simulink library "Garbage"  (R2023a)
settings: Solver=ode45  StartTime=0.0  StopTime=10.0  RelTol=1e-3  EnableMultiTasking=on
undefined symbols (not defined in the file or in its folder): c, ending_column_index, ending_row_index, framebuffer_16x1, framebuffer_2x2, framebuffer_4x4, framebuffer_8x8, HEIGHT, hot, POS_X, POS_Y, starting_column_index, starting_row_index, WIDTH
libraries: Memory_Blocks_Lib x459, simulink x235, simulink_extras x132, dspmtrx3 x116, Bit_Multiplexers_Lib x67, Bit_Demultiplexers_Lib x49, Byte_Multiplexers_Lib x40, Miscellaneous x38, Computer_Library x17, Decoders_Lib x14, commutil2 x12, Arithmetic_Operations_Lib x8
(part 7 of 26)

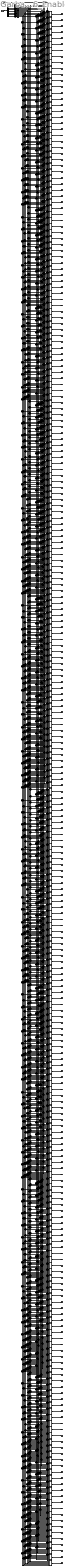
== system Garbage/Decoder_8_to_256_Enable ==
  Enable(1b) : Inport
  Addr(1B) : Inport  Port=2
  Lo`gical Operator{1,3,5..6,9} (5 alike) : Logic  Operator=NOT  IconShape=distinctive  AllPortsSameDT=off  OutDataTypeStr=boolean
  Logical Operator{1..81,84..111,113..146,148..243,246..262} (256 alike) : Logic  Inputs=9  IconShape=distinctive  AllPortsSameDT=off  OutDataTypeStr=boolean
  Logical Operator112 : Logic  Operator=NOT  IconShape=distinctive  AllPortsSameDT=off  OutDataTypeStr=boolean
  Logical Operator82 : Logic  Operator=NOT  IconShape=distinctive  AllPortsSameDT=off  OutDataTypeStr=boolean
  Logical Operator83 : Logic  Operator=NOT  IconShape=distinctive  AllPortsSameDT=off  OutDataTypeStr=boolean
  xyz1 : BusSelector  OutputSignals=signal1,signal2,signal3,signal4,signal5,signal6,signal7,signal8
  Select_255(1b) : Outport
  Select_254(1b) : Outport  Port=2
  Select_253(1b) : Outport  Port=3
  Select_252(1b) : Outport  Port=4
  Select_251(1b) : Outport  Port=5
  Select_250(1b) : Outport  Port=6
  Select_249(1b) : Outport  Port=7
  Select_248(1b) : Outport  Port=8
  Select_247(1b) : Outport  Port=9
  Select_246(1b) : Outport  Port=10
  Select_245(1b) : Outport  Port=11
  Select_244(1b) : Outport  Port=12
  Select_243(1b) : Outport  Port=13
  Select_242(1b) : Outport  Port=14
  Select_241(1b) : Outport  Port=15
  Select_240(1b) : Outport  Port=16
  Select_239(1b) : Outport  Port=17
  Select_238(1b) : Outport  Port=18
  Select_237(1b) : Outport  Port=19
  Select_236(1b) : Outport  Port=20
  Select_235(1b) : Outport  Port=21
  Select_234(1b) : Outport  Port=22
  Select_233(1b) : Outport  Port=23
  Select_232(1b) : Outport  Port=24
  Select_231(1b) : Outport  Port=25
  Select_230(1b) : Outport  Port=26
  Select_229(1b) : Outport  Port=27
  Select_228(1b) : Outport  Port=28
  Select_227(1b) : Outport  Port=29
  Select_226(1b) : Outport  Port=30
  Select_225(1b) : Outport  Port=31
  Select_224(1b) : Outport  Port=32
  Select_223(1b) : Outport  Port=33
  Select_222(1b) : Outport  Port=34
  Select_221(1b) : Outport  Port=35
  Select_220(1b) : Outport  Port=36
  Select_219(1b) : Outport  Port=37
  Select_218(1b) : Outport  Port=38
  Select_217(1b) : Outport  Port=39
  Select_216(1b) : Outport  Port=40
  Select_215(1b) : Outport  Port=41
  Select_214(1b) : Outport  Port=42
  Select_213(1b) : Outport  Port=43
  Select_212(1b) : Outport  Port=44
  Select_211(1b) : Outport  Port=45
  Select_210(1b) : Outport  Port=46
  Select_209(1b) : Outport  Port=47
  Select_208(1b) : Outport  Port=48
  Select_207(1b) : Outport  Port=49
  Select_206(1b) : Outport  Port=50
  Select_205(1b) : Outport  Port=51
  Select_204(1b) : Outport  Port=52
  Select_203(1b) : Outport  Port=53
  Select_202(1b) : Outport  Port=54
  Select_201(1b) : Outport  Port=55
  Select_200(1b) : Outport  Port=56
  Select_199(1b) : Outport  Port=57
  Select_198(1b) : Outport  Port=58
  Select_197(1b) : Outport  Port=59
  Select_196(1b) : Outport  Port=60
  Select_195(1b) : Outport  Port=61
  Select_194(1b) : Outport  Port=62
  Select_193(1b) : Outport  Port=63
  Select_192(1b) : Outport  Port=64
  Select_191(1b) : Outport  Port=65
  Select_190(1b) : Outport  Port=66
  Select_189(1b) : Outport  Port=67
  Select_188(1b) : Outport  Port=68
  Select_187(1b) : Outport  Port=69
  Select_186(1b) : Outport  Port=70
  Select_185(1b) : Outport  Port=71
  Select_184(1b) : Outport  Port=72
  Select_183(1b) : Outport  Port=73
  Select_182(1b) : Outport  Port=74
  Select_181(1b) : Outport  Port=75
  Select_180(1b) : Outport  Port=76
  Select_179(1b) : Outport  Port=77
  Select_178(1b) : Outport  Port=78
  Select_177(1b) : Outport  Port=79
  Select_176(1b) : Outport  Port=80
  Select_175(1b) : Outport  Port=81
  Select_174(1b) : Outport  Port=82
  Select_173(1b) : Outport  Port=83
  Select_172(1b) : Outport  Port=84
  Select_171(1b) : Outport  Port=85
  Select_170(1b) : Outport  Port=86
  Select_169(1b) : Outport  Port=87
  Select_168(1b) : Outport  Port=88
  Select_167(1b) : Outport  Port=89
  Select_166(1b) : Outport  Port=90
  Select_165(1b) : Outport  Port=91
  Select_164(1b) : Outport  Port=92
  Select_163(1b) : Outport  Port=93
  Select_162(1b) : Outport  Port=94
  Select_161(1b) : Outport  Port=95
  Select_160(1b) : Outport  Port=96
  Select_159(1b) : Outport  Port=97
  Select_158(1b) : Outport  Port=98
  Select_157(1b) : Outport  Port=99
  Select_156(1b) : Outport  Port=100
  Select_155(1b) : Outport  Port=101
  Select_154(1b) : Outport  Port=102
  Select_153(1b) : Outport  Port=103
  Select_152(1b) : Outport  Port=104
  Select_151(1b) : Outport  Port=105
  Select_150(1b) : Outport  Port=106
  Select_149(1b) : Outport  Port=107
  Select_148(1b) : Outport  Port=108
  Select_147(1b) : Outport  Port=109
  Select_146(1b) : Outport  Port=110
  Select_145(1b) : Outport  Port=111
  Select_144(1b) : Outport  Port=112
  Select_143(1b) : Outport  Port=113
  Select_142(1b) : Outport  Port=114
  Select_141(1b) : Outport  Port=115
  Select_140(1b) : Outport  Port=116
  Select_139(1b) : Outport  Port=117
  Select_138(1b) : Outport  Port=118
  Select_137(1b) : Outport  Port=119
  Select_136(1b) : Outport  Port=120
  Select_135(1b) : Outport  Port=121
  Select_134(1b) : Outport  Port=122
  Select_133(1b) : Outport  Port=123
  Select_132(1b) : Outport  Port=124
  Select_131(1b) : Outport  Port=125
  Select_130(1b) : Outport  Port=126
  Select_129(1b) : Outport  Port=127
  Select_128(1b) : Outport  Port=128
  Select_127(1b) : Outport  Port=129
  Select_126(1b) : Outport  Port=130
  Select_125(1b) : Outport  Port=131
  Select_124(1b) : Outport  Port=132
  Select_123(1b) : Outport  Port=133
  Select_122(1b) : Outport  Port=134
  Select_121(1b) : Outport  Port=135
  Select_120(1b) : Outport  Port=136
  Select_119(1b) : Outport  Port=137
  Select_118(1b) : Outport  Port=138
  Select_117(1b) : Outport  Port=139
  Select_116(1b) : Outport  Port=140
  Select_115(1b) : Outport  Port=141
  Select_114(1b) : Outport  Port=142
  Select_113(1b) : Outport  Port=143
  Select_112(1b) : Outport  Port=144
  Select_111(1b) : Outport  Port=145
  Select_110(1b) : Outport  Port=146
  Select_109(1b) : Outport  Port=147
  Select_108(1b) : Outport  Port=148
  Select_107(1b) : Outport  Port=149
  Select_106(1b) : Outport  Port=150
  Select_105(1b) : Outport  Port=151
  Select_104(1b) : Outport  Port=152
  Select_103(1b) : Outport  Port=153
  Select_102(1b) : Outport  Port=154
  Select_101(1b) : Outport  Port=155
  Select_100(1b) : Outport  Port=156
  Select_099(1b) : Outport  Port=157
  Select_098(1b) : Outport  Port=158
  Select_097(1b) : Outport  Port=159
  Select_096(1b) : Outport  Port=160
  Select_095(1b) : Outport  Port=161
  Select_094(1b) : Outport  Port=162
  Select_093(1b) : Outport  Port=163
  Select_092(1b) : Outport  Port=164
  Select_091(1b) : Outport  Port=165
  Select_090(1b) : Outport  Port=166
  Select_089(1b) : Outport  Port=167
  Select_088(1b) : Outport  Port=168
  Select_087(1b) : Outport  Port=169
  Select_086(1b) : Outport  Port=170
  Select_085(1b) : Outport  Port=171
  Select_084(1b) : Outport  Port=172
  Select_083(1b) : Outport  Port=173
  Select_082(1b) : Outport  Port=174
  Select_081(1b) : Outport  Port=175
  Select_080(1b) : Outport  Port=176
  Select_079(1b) : Outport  Port=177
  Select_078(1b) : Outport  Port=178
  Select_077(1b) : Outport  Port=179
  Select_076(1b) : Outport  Port=180
  Select_075(1b) : Outport  Port=181
  Select_074(1b) : Outport  Port=182
  Select_073(1b) : Outport  Port=183
  Select_072(1b) : Outport  Port=184
  Select_071(1b) : Outport  Port=185
  Select_070(1b) : Outport  Port=186
  Select_069(1b) : Outport  Port=187
  Select_068(1b) : Outport  Port=188
  Select_067(1b) : Outport  Port=189
  Select_066(1b) : Outport  Port=190
  Select_065(1b) : Outport  Port=191
  Select_064(1b) : Outport  Port=192
  Select_063(1b) : Outport  Port=193
  Select_062(1b) : Outport  Port=194
  Select_061(1b) : Outport  Port=195
  Select_060(1b) : Outport  Port=196
  Select_059(1b) : Outport  Port=197
  Select_058(1b) : Outport  Port=198
  Select_057(1b) : Outport  Port=199
  Select_056(1b) : Outport  Port=200
  Select_055(1b) : Outport  Port=201
  Select_054(1b) : Outport  Port=202
  Select_053(1b) : Outport  Port=203
  Select_052(1b) : Outport  Port=204
  Select_051(1b) : Outport  Port=205
  Select_050(1b) : Outport  Port=206
  Select_049(1b) : Outport  Port=207
  Select_048(1b) : Outport  Port=208
  Select_047(1b) : Outport  Port=209
  Select_046(1b) : Outport  Port=210
  Select_045(1b) : Outport  Port=211
  Select_044(1b) : Outport  Port=212
  Select_043(1b) : Outport  Port=213
  Select_042(1b) : Outport  Port=214
  Select_041(1b) : Outport  Port=215
  Select_040(1b) : Outport  Port=216
  Select_039(1b) : Outport  Port=217
  Select_038(1b) : Outport  Port=218
  Select_037(1b) : Outport  Port=219
  Select_036(1b) : Outport  Port=220
  Select_035(1b) : Outport  Port=221
  Select_034(1b) : Outport  Port=222
  Select_033(1b) : Outport  Port=223
  Select_032(1b) : Outport  Port=224
  Select_031(1b) : Outport  Port=225
  Select_030(1b) : Outport  Port=226
  Select_029(1b) : Outport  Port=227
  Select_028(1b) : Outport  Port=228
  Select_027(1b) : Outport  Port=229
  Select_026(1b) : Outport  Port=230
  Select_025(1b) : Outport  Port=231
  Select_024(1b) : Outport  Port=232
  Select_023(1b) : Outport  Port=233
  Select_022(1b) : Outport  Port=234
  Select_021(1b) : Outport  Port=235
  Select_020(1b) : Outport  Port=236
  Select_019(1b) : Outport  Port=237
  Select_018(1b) : Outport  Port=238
  Select_017(1b) : Outport  Port=239
  Select_016(1b) : Outport  Port=240
  Select_015(1b) : Outport  Port=241
  Select_014(1b) : Outport  Port=242
  Select_013(1b) : Outport  Port=243
  Select_012(1b) : Outport  Port=244
  Select_011(1b) : Outport  Port=245
  Select_010(1b) : Outport  Port=246
  Select_009(1b) : Outport  Port=247
  Select_008(1b) : Outport  Port=248
  Select_007(1b) : Outport  Port=249
  Select_006(1b) : Outport  Port=250
  Select_005(1b) : Outport  Port=251
  Select_004(1b) : Outport  Port=252
  Select_003(1b) : Outport  Port=253
  Select_002(1b) : Outport  Port=254
  Select_001(1b) : Outport  Port=255
  Select_000(1b) : Outport  Port=256
  wires:
  Logical Operator16/1 -> Select_176(1b)/1
  Logical Operator15/1 -> Select_177(1b)/1
  Logical Operator14/1 -> Select_178(1b)/1
  Logical Operator13/1 -> Select_179(1b)/1
  Logical Operator12/1 -> Select_180(1b)/1
  Logical Operator11/1 -> Select_181(1b)/1
  Logical Operator10/1 -> Select_182(1b)/1
  Logical Operator9/1 -> Select_183(1b)/1
  Logical Operator8/1 -> Select_184(1b)/1
  Logical Operator7/1 -> Select_185(1b)/1
  Logical Operator6/1 -> Select_186(1b)/1
  Logical Operator4/1 -> Select_187(1b)/1
  Logical Operator1/1 -> Select_190(1b)/1
  Logical Operator2/1 -> Select_189(1b)/1
  Logical Operator3/1 -> Select_188(1b)/1
  Logical Operator63/1 -> Select_191(1b)/1
  Logical Operator26/1 -> Select_160(1b)/1
  Logical Operator25/1 -> Select_161(1b)/1
  Logical Operator24/1 -> Select_162(1b)/1
  Logical Operator21/1 -> Select_163(1b)/1
  Logical Operator20/1 -> Select_164(1b)/1
  Logical Operator19/1 -> Select_165(1b)/1
  Logical Operator18/1 -> Select_166(1b)/1
  Logical Operator34/1 -> Select_167(1b)/1
  Logical Operator33/1 -> Select_168(1b)/1
  Logical Operator32/1 -> Select_169(1b)/1
  Logical Operator30/1 -> Select_170(1b)/1
  Logical Operator29/1 -> Select_171(1b)/1
  Logical Operator17/1 -> Select_174(1b)/1
  Logical Operator27/1 -> Select_173(1b)/1
  Logical Operator28/1 -> Select_172(1b)/1
  Logical Operator31/1 -> Select_175(1b)/1
  Logical Operator42/1 -> Select_144(1b)/1
  Logical Operator41/1 -> Select_145(1b)/1
  Logical Operator40/1 -> Select_146(1b)/1
  Logical Operator39/1 -> Select_147(1b)/1
  Logical Operator38/1 -> Select_148(1b)/1
  Logical Operator37/1 -> Select_149(1b)/1
  Logical Operator36/1 -> Select_150(1b)/1
  Logical Operator50/1 -> Select_151(1b)/1
  Logical Operator49/1 -> Select_152(1b)/1
  Logical Operator48/1 -> Select_153(1b)/1
  Logical Operator46/1 -> Select_154(1b)/1
  Logical Operator45/1 -> Select_155(1b)/1
  Logical Operator35/1 -> Select_158(1b)/1
  Logical Operator43/1 -> Select_157(1b)/1
  Logical Operator44/1 -> Select_156(1b)/1
  Logical Operator47/1 -> Select_159(1b)/1
  Logical Operator58/1 -> Select_128(1b)/1
  Logical Operator57/1 -> Select_129(1b)/1
  Logical Operator56/1 -> Select_130(1b)/1
  Logical Operator55/1 -> Select_131(1b)/1
  Logical Operator54/1 -> Select_132(1b)/1
  Logical Operator53/1 -> Select_133(1b)/1
  Logical Operator52/1 -> Select_134(1b)/1
  Logical Operator67/1 -> Select_135(1b)/1
  Logical Operator66/1 -> Select_136(1b)/1
  Logical Operator65/1 -> Select_137(1b)/1
  Logical Operator62/1 -> Select_138(1b)/1
  Logical Operator61/1 -> Select_139(1b)/1
  Logical Operator51/1 -> Select_142(1b)/1
  Logical Operator59/1 -> Select_141(1b)/1
  Logical Operator60/1 -> Select_140(1b)/1
  Logical Operator64/1 -> Select_143(1b)/1
  xyz1/1 -> Logical Operator68/2, Logical Operator79/2, Logical Operator90/2, Logical Operator101/2, Logical Operator123/2, Logical Operator132/2, Logical Operator133/2, Logical Operator134/2, Logical Operator69/2, Logical Operator70/2, Logical Operator71/2, Logical Operator72/2, Logical Operator73/2, Logical Operator74/2, Logical Operator75/2, Logical Operator92/2, Logical Operator76/2, Logical Operator87/2, Logical Operator88/2, Logical Operator89/2, Logical Operator91/2, Logical Operator93/2, Logical Operator94/2, Logical Operator95/2, Logical Operator77/2, Logical Operator78/2, Logical Operator80/2, Logical Operator81/2, Logical Operator84/2, Logical Operator85/2, Logical Operator86/2, Logical Operator109/2, Logical Operator96/2, Logical Operator105/2, Logical Operator106/2, Logical Operator107/2, Logical Operator108/2, Logical Operator110/2, Logical Operator111/2, Logical Operator113/2, Logical Operator97/2, Logical Operator98/2, Logical Operator99/2, Logical Operator100/2, Logical Operator102/2, Logical Operator103/2, Logical Operator104/2, Logical Operator128/2, Logical Operator114/2, Logical Operator122/2, Logical Operator124/2, Logical Operator125/2, Logical Operator126/2, Logical Operator129/2, Logical Operator130/2, Logical Operator131/2, Logical Operator115/2, Logical Operator116/2, Logical Operator117/2, Logical Operator118/2, Logical Operator119/2, Logical Operator120/2, Logical Operator121/2, Logical Operator63/2, Logical Operator1/2, Logical Operator2/2, Logical Operator3/2, Logical Operator4/2, Logical Operator6/2, Logical Operator7/2, Logical Operator8/2, Logical Operator9/2, Logical Operator10/2, Logical Operator11/2, Logical Operator12/2, Logical Operator13/2, Logical Operator14/2, Logical Operator15/2, Logical Operator16/2, Logical Operator31/2, Logical Operator17/2, Logical Operator27/2, Logical Operator28/2, Logical Operator29/2, Logical Operator30/2, Logical Operator32/2, Logical Operator33/2, Logical Operator34/2, Logical Operator18/2, Logical Operator19/2, Logical Operator20/2, Logical Operator21/2, Logical Operator24/2, Logical Operator25/2, Logical Operator26/2, Logical Operator47/2, Logical Operator35/2, Logical Operator43/2, Logical Operator44/2, Logical Operator45/2, Logical Operator46/2, Logical Operator48/2, Logical Operator49/2, Logical Operator50/2, Logical Operator36/2, Logical Operator37/2, Logical Operator38/2, Logical Operator39/2, Logical Operator40/2, Logical Operator41/2, Logical Operator42/2, Logical Operator64/2, Logical Operator51/2, Logical Operator59/2, Logical Operator60/2, Logical Operator61/2, Logical Operator62/2, Logical Operator65/2, Logical Operator66/2, Logical Operator67/2, Logical Operator52/2, Logical Operator53/2, Logical Operator54/2, Logical Operator55/2, Logical Operator56/2, Logical Operator57/2, Logical Operator58/2, Logical Operator127/2, Lo`gical Operator9/1  "<signal1>"
  Logical Operator75/1 -> Select_240(1b)/1
  Logical Operator74/1 -> Select_241(1b)/1
  Logical Operator73/1 -> Select_242(1b)/1
  Logical Operator72/1 -> Select_243(1b)/1
  Logical Operator71/1 -> Select_244(1b)/1
  Logical Operator70/1 -> Select_245(1b)/1
  Logical Operator69/1 -> Select_246(1b)/1
  Logical Operator134/1 -> Select_247(1b)/1
  Logical Operator133/1 -> Select_248(1b)/1
  Logical Operator132/1 -> Select_249(1b)/1
  Logical Operator123/1 -> Select_250(1b)/1
  Logical Operator101/1 -> Select_251(1b)/1
  Logical Operator68/1 -> Select_254(1b)/1
  Logical Operator79/1 -> Select_253(1b)/1
  Logical Operator90/1 -> Select_252(1b)/1
  Logical Operator127/1 -> Select_255(1b)/1
  Logical Operator86/1 -> Select_224(1b)/1
  Logical Operator85/1 -> Select_225(1b)/1
  Logical Operator84/1 -> Select_226(1b)/1
  Logical Operator81/1 -> Select_227(1b)/1
  Logical Operator80/1 -> Select_228(1b)/1
  Logical Operator78/1 -> Select_229(1b)/1
  Logical Operator77/1 -> Select_230(1b)/1
  Logical Operator95/1 -> Select_231(1b)/1
  Logical Operator94/1 -> Select_232(1b)/1
  Logical Operator93/1 -> Select_233(1b)/1
  Logical Operator91/1 -> Select_234(1b)/1
  Logical Operator89/1 -> Select_235(1b)/1
  Logical Operator76/1 -> Select_238(1b)/1
  Logical Operator87/1 -> Select_237(1b)/1
  Logical Operator88/1 -> Select_236(1b)/1
  Logical Operator92/1 -> Select_239(1b)/1
  Logical Operator104/1 -> Select_208(1b)/1
  Logical Operator103/1 -> Select_209(1b)/1
  Logical Operator102/1 -> Select_210(1b)/1
  Logical Operator100/1 -> Select_211(1b)/1
  Logical Operator99/1 -> Select_212(1b)/1
  Logical Operator98/1 -> Select_213(1b)/1
  Logical Operator97/1 -> Select_214(1b)/1
  Logical Operator113/1 -> Select_215(1b)/1
  Logical Operator111/1 -> Select_216(1b)/1
  Logical Operator110/1 -> Select_217(1b)/1
  Logical Operator108/1 -> Select_218(1b)/1
  Logical Operator107/1 -> Select_219(1b)/1
  Logical Operator96/1 -> Select_222(1b)/1
  Logical Operator105/1 -> Select_221(1b)/1
  Logical Operator106/1 -> Select_220(1b)/1
  Logical Operator109/1 -> Select_223(1b)/1
  Logical Operator121/1 -> Select_192(1b)/1
  Logical Operator120/1 -> Select_193(1b)/1
  Logical Operator119/1 -> Select_194(1b)/1
  Logical Operator118/1 -> Select_195(1b)/1
  Logical Operator117/1 -> Select_196(1b)/1
  Logical Operator116/1 -> Select_197(1b)/1
  Logical Operator115/1 -> Select_198(1b)/1
  Logical Operator131/1 -> Select_199(1b)/1
  Logical Operator130/1 -> Select_200(1b)/1
  Logical Operator129/1 -> Select_201(1b)/1
  Logical Operator126/1 -> Select_202(1b)/1
  Logical Operator125/1 -> Select_203(1b)/1
  Logical Operator114/1 -> Select_206(1b)/1
  Logical Operator122/1 -> Select_205(1b)/1
  Logical Operator124/1 -> Select_204(1b)/1
  Logical Operator128/1 -> Select_207(1b)/1
  Logical Operator174/1 -> Select_048(1b)/1
  Logical Operator173/1 -> Select_049(1b)/1
  Logical Operator172/1 -> Select_050(1b)/1
  Logical Operator166/1 -> Select_051(1b)/1
  Logical Operator155/1 -> Select_052(1b)/1
  Logical Operator144/1 -> Select_053(1b)/1
  Logical Operator22/1 -> Select_054(1b)/1
  Logical Operator252/1 -> Select_055(1b)/1
  Logical Operator241/1 -> Select_056(1b)/1
  Logical Operator230/1 -> Select_057(1b)/1
  Logical Operator219/1 -> Select_058(1b)/1
  Logical Operator198/1 -> Select_059(1b)/1
  Logical Operator5/1 -> Select_062(1b)/1
  Logical Operator178/1 -> Select_061(1b)/1
  Logical Operator187/1 -> Select_060(1b)/1
  Logical Operator223/1 -> Select_063(1b)/1
  Logical Operator183/1 -> Select_032(1b)/1
  Logical Operator182/1 -> Select_033(1b)/1
  Logical Operator181/1 -> Select_034(1b)/1
  Logical Operator180/1 -> Select_035(1b)/1
  Logical Operator179/1 -> Select_036(1b)/1
  Logical Operator177/1 -> Select_037(1b)/1
  Logical Operator176/1 -> Select_038(1b)/1
  Logical Operator192/1 -> Select_039(1b)/1
  Logical Operator191/1 -> Select_040(1b)/1
  Logical Operator190/1 -> Select_041(1b)/1
  Logical Operator188/1 -> Select_042(1b)/1
  Logical Operator186/1 -> Select_043(1b)/1
  Logical Operator175/1 -> Select_046(1b)/1
  Logical Operator184/1 -> Select_045(1b)/1
  Logical Operator185/1 -> Select_044(1b)/1
  Logical Operator189/1 -> Select_047(1b)/1
  xyz1/3 -> Lo`gical Operator5/1, Logical Operator127/4, Logical Operator68/4, Logical Operator79/4, Logical Operator90/4, Logical Operator101/4, Logical Operator123/4, Logical Operator132/4, Logical Operator133/4, Logical Operator134/4, Logical Operator69/4, Logical Operator70/4, Logical Operator71/4, Logical Operator72/4, Logical Operator73/4, Logical Operator74/4, Logical Operator75/4, Logical Operator92/4, Logical Operator76/4, Logical Operator87/4, Logical Operator88/4, Logical Operator89/4, Logical Operator91/4, Logical Operator93/4, Logical Operator94/4, Logical Operator95/4, Logical Operator77/4, Logical Operator78/4, Logical Operator80/4, Logical Operator81/4, Logical Operator84/4, Logical Operator85/4, Logical Operator86/4, Logical Operator63/4, Logical Operator1/4, Logical Operator2/4, Logical Operator3/4, Logical Operator4/4, Logical Operator6/4, Logical Operator7/4, Logical Operator8/4, Logical Operator9/4, Logical Operator10/4, Logical Operator11/4, Logical Operator12/4, Logical Operator13/4, Logical Operator14/4, Logical Operator15/4, Logical Operator16/4, Logical Operator31/4, Logical Operator17/4, Logical Operator27/4, Logical Operator28/4, Logical Operator29/4, Logical Operator30/4, Logical Operator32/4, Logical Operator33/4, Logical Operator34/4, Logical Operator18/4, Logical Operator19/4, Logical Operator20/4, Logical Operator21/4, Logical Operator24/4, Logical Operator163/4, Logical Operator228/4, Logical Operator240/4, Logical Operator253/4, Logical Operator135/4, Logical Operator159/4, Logical Operator169/4, Logical Operator170/4, Logical Operator171/4, Logical Operator229/4, Logical Operator231/4, Logical Operator232/4, Logical Operator233/4, Logical Operator234/4, Logical Operator235/4, Logical Operator236/4, Logical Operator255/4, Logical Operator237/4, Logical Operator249/4, Logical Operator250/4, Logical Operator251/4, Logical Operator254/4, Logical Operator256/4, Logical Operator257/4, Logical Operator258/4, Logical Operator238/4, Logical Operator239/4, Logical Operator242/4, Logical Operator243/4, Logical Operator246/4, Logical Operator247/4, Logical Operator248/4, Logical Operator223/4, Logical Operator5/4, Logical Operator178/4, Logical Operator187/4, Logical Operator198/4, Logical Operator219/4, Logical Operator230/4, Logical Operator241/4, Logical Operator252/4, Logical Operator22/4, Logical Operator144/4, Logical Operator155/4, Logical Operator166/4, Logical Operator172/4, Logical Operator173/4, Logical Operator174/4, Logical Operator189/4, Logical Operator175/4, Logical Operator184/4, Logical Operator185/4, Logical Operator186/4, Logical Operator188/4, Logical Operator190/4, Logical Operator191/4, Logical Operator192/4, Logical Operator176/4, Logical Operator177/4, Logical Operator179/4, Logical Operator180/4, Logical Operator181/4, Logical Operator183/4, Logical Operator182/4, Logical Operator26/4, Logical Operator25/4  "<signal3>"
  Logical Operator201/1 -> Select_016(1b)/1
  Logical Operator200/1 -> Select_017(1b)/1
  Logical Operator199/1 -> Select_018(1b)/1
  Logical Operator197/1 -> Select_019(1b)/1
  Logical Operator196/1 -> Select_020(1b)/1
  Logical Operator195/1 -> Select_021(1b)/1
  Logical Operator194/1 -> Select_022(1b)/1
  Logical Operator209/1 -> Select_023(1b)/1
  Logical Operator208/1 -> Select_024(1b)/1
  Logical Operator207/1 -> Select_025(1b)/1
  Logical Operator205/1 -> Select_026(1b)/1
  Logical Operator204/1 -> Select_027(1b)/1
  Logical Operator193/1 -> Select_030(1b)/1
  Logical Operator202/1 -> Select_029(1b)/1
  Logical Operator203/1 -> Select_028(1b)/1
  Logical Operator206/1 -> Select_031(1b)/1
  Logical Operator82/1 -> Logical Operator79/8, Logical Operator90/8, Logical Operator132/8, Logical Operator133/8, Logical Operator70/8, Logical Operator71/8, Logical Operator74/8, Logical Operator75/8, Logical Operator87/8, Logical Operator88/8, Logical Operator93/8, Logical Operator94/8, Logical Operator78/8, Logical Operator80/8, Logical Operator85/8, Logical Operator86/8, Logical Operator105/8, Logical Operator106/8, Logical Operator110/8, Logical Operator111/8, Logical Operator98/8, Logical Operator99/8, Logical Operator103/8, Logical Operator104/8, Logical Operator122/8, Logical Operator124/8, Logical Operator129/8, Logical Operator130/8, Logical Operator116/8, Logical Operator117/8, Logical Operator120/8, Logical Operator121/8, Logical Operator2/8, Logical Operator3/8, Logical Operator7/8, Logical Operator8/8, Logical Operator11/8, Logical Operator12/8, Logical Operator15/8, Logical Operator16/8, Logical Operator27/8, Logical Operator28/8, Logical Operator32/8, Logical Operator33/8, Logical Operator19/8, Logical Operator20/8, Logical Operator25/8, Logical Operator26/8, Logical Operator43/8, Logical Operator44/8, Logical Operator48/8, Logical Operator49/8, Logical Operator37/8, Logical Operator38/8, Logical Operator41/8, Logical Operator42/8, Logical Operator59/8, Logical Operator60/8, Logical Operator65/8, Logical Operator66/8, Logical Operator53/8, Logical Operator54/8, Logical Operator240/8, Logical Operator253/8, Logical Operator169/8, Logical Operator170/8, Logical Operator231/8, Logical Operator232/8, Logical Operator235/8, Logical Operator236/8, Logical Operator249/8, Logical Operator250/8, Logical Operator256/8, Logical Operator257/8, Logical Operator239/8, Logical Operator242/8, Logical Operator247/8, Logical Operator248/8, Logical Operator139/8, Logical Operator140/8, Logical Operator145/8, Logical Operator146/8, Logical Operator261/8, Logical Operator262/8, Logical Operator137/8, Logical Operator138/8, Logical Operator158/8, Logical Operator160/8, Logical Operator165/8, Logical Operator167/8, Logical Operator151/8, Logical Operator152/8, Logical Operator156/8, Logical Operator157/8, Logical Operator178/8, Logical Operator187/8, Logical Operator230/8, Logical Operator241/8, Logical Operator144/8, Logical Operator155/8, Logical Operator173/8, Logical Operator174/8, Logical Operator184/8, Logical Operator185/8, Logical Operator190/8, Logical Operator191/8, Logical Operator177/8, Logical Operator179/8, Logical Operator182/8, Logical Operator183/8, Logical Operator202/8, Logical Operator203/8, Logical Operator207/8, Logical Operator208/8, Logical Operator195/8, Logical Operator196/8, Logical Operator200/8, Logical Operator201/8, Logical Operator218/8, Logical Operator220/8, Logical Operator225/8, Logical Operator226/8, Logical Operator212/8, Logical Operator213/8, Logical Operator217/8, Logical Operator216/8, Logical Operator58/8, Logical Operator57/8
  xyz1/8 -> Logical Operator127/9, Logical Operator79/9, Logical Operator101/9, Logical Operator132/9, Logical Operator134/9, Logical Operator70/9, Logical Operator72/9, Logical Operator74/9, Logical Operator92/9, Logical Operator87/9, Logical Operator89/9, Logical Operator93/9, Logical Operator95/9, Logical Operator78/9, Logical Operator81/9, Logical Operator85/9, Logical Operator109/9, Logical Operator105/9, Logical Operator107/9, Logical Operator110/9, Logical Operator113/9, Logical Operator98/9, Logical Operator100/9, Logical Operator103/9, Logical Operator128/9, Logical Operator122/9, Logical Operator125/9, Logical Operator129/9, Logical Operator131/9, Logical Operator116/9, Logical Operator118/9, Logical Operator120/9, Logical Operator63/9, Logical Operator2/9, Logical Operator4/9, Logical Operator7/9, Logical Operator9/9, Logical Operator11/9, Logical Operator13/9, Logical Operator15/9, Logical Operator31/9, Logical Operator27/9, Logical Operator29/9, Logical Operator32/9, Logical Operator34/9, Logical Operator19/9, Logical Operator21/9, Logical Operator25/9, Logical Operator47/9, Logical Operator43/9, Logical Operator45/9, Logical Operator48/9, Logical Operator50/9, Logical Operator37/9, Logical Operator39/9, Logical Operator41/9, Logical Operator64/9, Logical Operator59/9, Logical Operator61/9, Logical Operator65/9, Logical Operator67/9, Logical Operator53/9, Logical Operator55/9, Logical Operator163/9, Logical Operator240/9, Logical Operator135/9, Logical Operator169/9, Logical Operator171/9, Logical Operator231/9, Logical Operator233/9, Logical Operator235/9, Logical Operator255/9, Logical Operator249/9, Logical Operator251/9, Logical Operator256/9, Logical Operator258/9, Logical Operator239/9, Logical Operator243/9, Logical Operator247/9, Logical Operator143/9, Logical Operator139/9, Logical Operator141/9, Logical Operator145/9, Logical Operator148/9, Logical Operator261/9, Logical Operator23/9, Logical Operator137/9, Logical Operator164/9, Logical Operator158/9, Logical Operator161/9, Logical Operator165/9, Logical Operator168/9, Logical Operator151/9, Logical Operator153/9, Logical Operator156/9, Logical Operator223/9, Logical Operator178/9, Logical Operator198/9, Logical Operator230/9, Logical Operator252/9, Logical Operator144/9, Logical Operator166/9, Logical Operator173/9, Logical Operator189/9, Logical Operator184/9, Logical Operator186/9, Logical Operator190/9, Logical Operator192/9, Logical Operator177/9, Logical Operator180/9, Logical Operator182/9, Logical Operator206/9, Logical Operator202/9, Logical Operator204/9, Logical Operator207/9, Logical Operator209/9, Logical Operator195/9, Logical Operator197/9, Logical Operator200/9, Logical Operator224/9, Logical Operator218/9, Logical Operator221/9, Logical Operator225/9, Logical Operator227/9, Logical Operator212/9, Logical Operator214/9, Logical Operator216/9, Logical Operator57/9, Logical Operator83/1  "<signal8>"
  Logical Operator83/1 -> Logical Operator68/9, Logical Operator90/9, Logical Operator123/9, Logical Operator133/9, Logical Operator69/9, Logical Operator71/9, Logical Operator73/9, Logical Operator75/9, Logical Operator76/9, Logical Operator88/9, Logical Operator91/9, Logical Operator94/9, Logical Operator77/9, Logical Operator80/9, Logical Operator84/9, Logical Operator86/9, Logical Operator96/9, Logical Operator106/9, Logical Operator108/9, Logical Operator111/9, Logical Operator97/9, Logical Operator99/9, Logical Operator102/9, Logical Operator104/9, Logical Operator114/9, Logical Operator124/9, Logical Operator126/9, Logical Operator130/9, Logical Operator115/9, Logical Operator117/9, Logical Operator119/9, Logical Operator121/9, Logical Operator1/9, Logical Operator3/9, Logical Operator6/9, Logical Operator8/9, Logical Operator10/9, Logical Operator12/9, Logical Operator14/9, Logical Operator16/9, Logical Operator17/9, Logical Operator28/9, Logical Operator30/9, Logical Operator33/9, Logical Operator18/9, Logical Operator20/9, Logical Operator24/9, Logical Operator26/9, Logical Operator35/9, Logical Operator44/9, Logical Operator46/9, Logical Operator49/9, Logical Operator36/9, Logical Operator38/9, Logical Operator40/9, Logical Operator42/9, Logical Operator51/9, Logical Operator60/9, Logical Operator62/9, Logical Operator66/9, Logical Operator52/9, Logical Operator54/9, Logical Operator56/9, Logical Operator228/9, Logical Operator253/9, Logical Operator159/9, Logical Operator170/9, Logical Operator229/9, Logical Operator232/9, Logical Operator234/9, Logical Operator236/9, Logical Operator237/9, Logical Operator250/9, Logical Operator254/9, Logical Operator257/9, Logical Operator238/9, Logical Operator242/9, Logical Operator246/9, Logical Operator248/9, Logical Operator259/9, Logical Operator140/9, Logical Operator142/9, Logical Operator146/9, Logical Operator260/9, Logical Operator262/9, Logical Operator136/9, Logical Operator138/9, Logical Operator149/9, Logical Operator160/9, Logical Operator162/9, Logical Operator167/9, Logical Operator150/9, Logical Operator152/9, Logical Operator154/9, Logical Operator157/9, Logical Operator5/9, Logical Operator187/9, Logical Operator219/9, Logical Operator241/9, Logical Operator22/9, Logical Operator155/9, Logical Operator172/9, Logical Operator174/9, Logical Operator175/9, Logical Operator185/9, Logical Operator188/9, Logical Operator191/9, Logical Operator176/9, Logical Operator179/9, Logical Operator181/9, Logical Operator183/9, Logical Operator193/9, Logical Operator203/9, Logical Operator205/9, Logical Operator208/9, Logical Operator194/9, Logical Operator196/9, Logical Operator199/9, Logical Operator201/9, Logical Operator210/9, Logical Operator220/9, Logical Operator222/9, Logical Operator226/9, Logical Operator211/9, Logical Operator213/9, Logical Operator215/9, Logical Operator217/9, Logical Operator58/9
  Logical Operator112/1 -> Logical Operator101/7, Logical Operator123/7, Logical Operator132/7, Logical Operator133/7, Logical Operator72/7, Logical Operator73/7, Logical Operator74/7, Logical Operator75/7, Logical Operator89/7, Logical Operator91/7, Logical Operator93/7, Logical Operator94/7, Logical Operator81/7, Logical Operator84/7, Logical Operator85/7, Logical Operator86/7, Logical Operator107/7, Logical Operator108/7, Logical Operator110/7, Logical Operator111/7, Logical Operator100/7, Logical Operator102/7, Logical Operator103/7, Logical Operator104/7, Logical Operator125/7, Logical Operator126/7, Logical Operator129/7, Logical Operator130/7, Logical Operator118/7, Logical Operator119/7, Logical Operator120/7, Logical Operator121/7, Logical Operator4/7, Logical Operator6/7, Logical Operator7/7, Logical Operator8/7, Logical Operator13/7, Logical Operator14/7, Logical Operator15/7, Logical Operator16/7, Logical Operator29/7, Logical Operator30/7, Logical Operator32/7, Logical Operator33/7, Logical Operator21/7, Logical Operator24/7, Logical Operator25/7, Logical Operator26/7, Logical Operator45/7, Logical Operator46/7, Logical Operator48/7, Logical Operator49/7, Logical Operator39/7, Logical Operator40/7, Logical Operator41/7, Logical Operator42/7, Logical Operator61/7, Logical Operator62/7, Logical Operator65/7, Logical Operator66/7, Logical Operator55/7, Logical Operator56/7, Logical Operator135/7, Logical Operator159/7, Logical Operator169/7, Logical Operator170/7, Logical Operator233/7, Logical Operator234/7, Logical Operator235/7, Logical Operator236/7, Logical Operator251/7, Logical Operator254/7, Logical Operator256/7, Logical Operator257/7, Logical Operator243/7, Logical Operator246/7, Logical Operator247/7, Logical Operator248/7, Logical Operator141/7, Logical Operator142/7, Logical Operator145/7, Logical Operator146/7, Logical Operator23/7, Logical Operator136/7, Logical Operator137/7, Logical Operator138/7, Logical Operator161/7, Logical Operator162/7, Logical Operator165/7, Logical Operator167/7, Logical Operator153/7, Logical Operator154/7, Logical Operator156/7, Logical Operator157/7, Logical Operator198/7, Logical Operator219/7, Logical Operator230/7, Logical Operator241/7, Logical Operator166/7, Logical Operator172/7, Logical Operator173/7, Logical Operator174/7, Logical Operator186/7, Logical Operator188/7, Logical Operator190/7, Logical Operator191/7, Logical Operator180/7, Logical Operator181/7, Logical Operator182/7, Logical Operator183/7, Logical Operator204/7, Logical Operator205/7, Logical Operator207/7, Logical Operator208/7, Logical Operator197/7, Logical Operator199/7, Logical Operator200/7, Logical Operator201/7, Logical Operator221/7, Logical Operator222/7, Logical Operator225/7, Logical Operator226/7, Logical Operator214/7, Logical Operator215/7, Logical Operator217/7, Logical Operator216/7, Logical Operator58/7, Logical Operator57/7
  xyz1/5 -> Lo`gical Operator6/1, Logical Operator127/6, Logical Operator68/6, Logical Operator79/6, Logical Operator90/6, Logical Operator101/6, Logical Operator123/6, Logical Operator132/6, Logical Operator133/6, Logical Operator92/6, Logical Operator76/6, Logical Operator87/6, Logical Operator88/6, Logical Operator89/6, Logical Operator91/6, Logical Operator93/6, Logical Operator94/6, Logical Operator109/6, Logical Operator96/6, Logical Operator105/6, Logical Operator106/6, Logical Operator107/6, Logical Operator108/6, Logical Operator110/6, Logical Operator111/6, Logical Operator128/6, Logical Operator114/6, Logical Operator122/6, Logical Operator124/6, Logical Operator125/6, Logical Operator126/6, Logical Operator129/6, Logical Operator130/6, Logical Operator63/6, Logical Operator1/6, Logical Operator2/6, Logical Operator3/6, Logical Operator4/6, Logical Operator6/6, Logical Operator7/6, Logical Operator8/6, Logical Operator31/6, Logical Operator17/6, Logical Operator27/6, Logical Operator28/6, Logical Operator29/6, Logical Operator30/6, Logical Operator32/6, Logical Operator33/6, Logical Operator47/6, Logical Operator35/6, Logical Operator43/6, Logical Operator44/6, Logical Operator45/6, Logical Operator46/6, Logical Operator48/6, Logical Operator49/6, Logical Operator64/6, Logical Operator51/6, Logical Operator59/6, Logical Operator60/6, Logical Operator61/6, Logical Operator62/6, Logical Operator66/6, Logical Operator163/6, Logical Operator228/6, Logical Operator240/6, Logical Operator253/6, Logical Operator135/6, Logical Operator159/6, Logical Operator169/6, Logical Operator170/6, Logical Operator255/6, Logical Operator237/6, Logical Operator249/6, Logical Operator250/6, Logical Operator251/6, Logical Operator254/6, Logical Operator256/6, Logical Operator257/6, Logical Operator143/6, Logical Operator259/6, Logical Operator139/6, Logical Operator140/6, Logical Operator141/6, Logical Operator142/6, Logical Operator145/6, Logical Operator146/6, Logical Operator164/6, Logical Operator149/6, Logical Operator158/6, Logical Operator160/6, Logical Operator161/6, Logical Operator162/6, Logical Operator165/6, Logical Operator167/6, Logical Operator223/6, Logical Operator5/6, Logical Operator178/6, Logical Operator187/6, Logical Operator198/6, Logical Operator219/6, Logical Operator230/6, Logical Operator241/6, Logical Operator189/6, Logical Operator175/6, Logical Operator184/6, Logical Operator185/6, Logical Operator186/6, Logical Operator188/6, Logical Operator190/6, Logical Operator191/6, Logical Operator206/6, Logical Operator193/6, Logical Operator202/6, Logical Operator203/6, Logical Operator204/6, Logical Operator205/6, Logical Operator207/6, Logical Operator208/6, Logical Operator224/6, Logical Operator210/6, Logical Operator218/6, Logical Operator220/6, Logical Operator221/6, Logical Operator222/6, Logical Operator226/6, Logical Operator225/6, Logical Operator65/6  "<signal5>"